simulink model "AcasXuClosedLoop_all"  (Simulink 10.0)
settings: Solver=VariableStepAuto  StartTime=0.0  StopTime=20  RelTol=1e-3
libraries: neural x14040, simulink x2
(part 13 of 534)

== system AcasXuClosedLoop_all/networks/Networks_m_2/AcasXu_5_2/Layer 5 ==
  "a{4} " : Inport  PortDimensions=50
      mask Display: e = 0.06;c1 = [120 230 180]/255;c2 = ([120 230 180]+0)/(256+128);c3 = ([120 230 180]+128)/(256+128);patch([0 1 1 0],[0 0 1 1],c1);patch([0 1 1 1-e 1-e 0+e],[0 0 1 1-e 0+e 0+e],c2);patch([0 0 1 1-e 0+e 0+e],[0 1 1 1-e 1-e 0+e],c3);plot([0 1 1 0 0],[0 0 1 1 0]);
  Delays 1 : Subsystem
      mask Display: e = 0.06;c1 = [120 150 230]/255;c2 = ([120 150 230]+0)/383;c3 = ([120 150 230]+128)/383;patch([0 1 1 0],[0 0 1 1],c1);patch([0 1 1 1-e 1-e 0+e],[0 0 1 1-e 0+e 0+e],c2);patch([0 0 1 1-e 0+e 0+e],[0 1 1 1-e 1-e 0+e],c3);plot([0 1 1 0 0],[0 0 1 1 0]);disp('0');
  "LW{5,4}" : Subsystem
      mask Display: e = 0.06;c1 = [120 150 230]/255;c2 = ([120 150 230]+0)/383;c3 = ([120 150 230]+128)/383;patch([0 1 1 0],[0 0 1 1],c1);patch([0 1 1 1-e 1-e 0+e],[0 0 1 1-e 0+e 0+e],c2);patch([0 0 1 1-e 0+e 0+e],[0 1 1 1-e 1-e 0+e],c3);plot([0 1 1 0 0],[0 0 1 1 0]);disp('W');
  "b{5}" : Constant  Value=[-0.913216999999999945458739603054709732532501220703125;0.09844060000000000310027559180525713600218296051025390625;1.1548899999999999721467247582040727138519287109375;-0.32563399999999997902477844036184251308441162109375;-0.1335260000000000057962523669630172662436962127685546875;0.341119000000000005545786052607581950724124908447265625;0.01802020000000000010675904604795505292713642120361328125;0.9002499999999999946709294817992486059665679931640625;0.18333399999999999696598251830437220633029937744140625;0.51134500000000004948930154569097794592380523681640625;-0.33117099999999999315747345463023521006107330322265625;0.8964060000000000361097818313282914459705352783203125;1.518850000000000033395508580724708735942840576171875;0.369159999999999988151699881200329400599002838134765625;-1.723759999999999958930629873066209256649017333984375;0.410592999999999985760723575367592275142669677734375;-0.186315000000000008384404281969182193279266357421875;0.265000999999999986567189580455305986106395721435546875;-0.1680520000000000069295680305003770627081394195556640625;0.198382000000000002781774810500792227685451507568359375;2.13187000000000015376144801848568022251129150390625;-0.058779299999999999604138878339654183946549892425537109375;0.64183900000000004837374945054762065410614013671875;-0.430126999999999981572074148061801679432392120361328125;0.022663200000000001399502735921487328596413135528564453125;-0.038571899999999999242294990153823164291679859161376953125;-0.1602760000000000017994494783124537207186222076416015625;0.32549499999999997879029933756100945174694061279296875;-0.49118600000000001148947603724082000553607940673828125;0.56853900000000001657696202528313733637332916259765625;-2.884020000000000027995383788947947323322296142578125;0.540777999999999980929032972198911011219024658203125;0.77833300000000005258726787360501475632190704345703125;-0.85023800000000004928324415232054889202117919921875;0.88560099999999997155697428752318955957889556884765625;-1.1629199999999999537436679020174778997898101806640625;-0.4910769999999999857465127206523902714252471923828125;0.689135999999999970810904414975084364414215087890625;-0.56418400000000001881517164292745292186737060546875;0.2479699999999999959765517587584326975047588348388671875;-0.38862900000000000222399876292911358177661895751953125;-0.04462950000000000250199860829525277949869632720947265625;0.4766340000000000021174173525650985538959503173828125;0.10841499999999999748201418015014496631920337677001953125;-1.9298900000000001053734877132228575646877288818359375;-1.2899700000000000610356210017926059663295745849609375;0.192620999999999986673770990819321013987064361572265625;-0.24735099999999998754418584212544374167919158935546875;0.300580000000000013837819778927951119840145111083984375;0.1147660000000000068975936073911725543439388275146484375]
      mask Display: e = 0.06;c1 = [120 150 230]/255;c2 = ([120 150 230]+0)/383;c3 = ([120 150 230]+128)/383;patch([0 1 1 0],[0 0 1 1],c1);patch([0 1 1 1-e 1-e 0+e],[0 0 1 1-e 0+e 0+e],c2);patch([0 0 1 1-e 0+e 0+e],[0 1 1 1-e 1-e 0+e],c3);plot([0 1 1 0 0],[0 0 1 1 0]);disp('b');
  netsum : Sum  Inputs=2  SaturateOnIntegerOverflow=on  InputSameDT=on  OutDataTypeStr=Inherit: Same as first input
      mask Display: e = 0.06;c1 = [120 150 230]/255;c2 = ([120 150 230]+0)/383;c3 = ([120 150 230]+128)/383;patch([0 1 1 0],[0 0 1 1],c1);patch([0 1 1 1-e 1-e 0+e],[0 0 1 1-e 0+e 0+e],c2);patch([0 0 1 1-e 0+e 0+e],[0 1 1 1-e 1-e 0+e],c3);plot([0 1 1 0 0],[0 0 1 1 0]);disp('+');
  poslin : neural/Transfer Functions/poslin
      settings: SourceProductName=Deep Learning Toolbox  SourceProductBaseCode=NN
  "a{5}" : Outport  InitialOutput=[0;0;0;0;0;0;0;0;0;0;0;0;0;0;0;0;0;0;0;0;0;0;0;0;0;0;0;0;0;0;0;0;0;0;0;0;0;0;0;0;0;0;0;0;0;0;0;0;0;0]
      mask Display: e = 0.06;c1 = [120 230 180]/255;c2 = ([120 230 180]+0)/(256+128);c3 = ([120 230 180]+128)/(256+128);patch([0 1 1 0],[0 0 1 1],c1);patch([0 1 1 1-e 1-e 0+e],[0 0 1 1-e 0+e 0+e],c2);patch([0 0 1 1-e 0+e 0+e],[0 1 1 1-e 1-e 0+e],c3);plot([0 1 1 0 0],[0 0 1 1 0]);
  wires:
  poslin/1 -> "a{5}"/1
  netsum/1 -> poslin/1
  "b{5}"/1 -> netsum/2
  "LW{5,4}"/1("lz{5,4}") -> netsum/1
  Delays 1/1("pd{5,4}") -> "LW{5,4}"/1("ad{5,4}")
  "a{4} "/1 -> Delays 1/1("p{5}")

== system AcasXuClosedLoop_all/networks/Networks_m_2/AcasXu_5_2/Layer 6 ==
  "a{5} " : Inport  PortDimensions=50
      mask Display: e = 0.06;c1 = [120 230 180]/255;c2 = ([120 230 180]+0)/(256+128);c3 = ([120 230 180]+128)/(256+128);patch([0 1 1 0],[0 0 1 1],c1);patch([0 1 1 1-e 1-e 0+e],[0 0 1 1-e 0+e 0+e],c2);patch([0 0 1 1-e 0+e 0+e],[0 1 1 1-e 1-e 0+e],c3);plot([0 1 1 0 0],[0 0 1 1 0]);
  Delays 1 : Subsystem
      mask Display: e = 0.06;c1 = [120 150 230]/255;c2 = ([120 150 230]+0)/383;c3 = ([120 150 230]+128)/383;patch([0 1 1 0],[0 0 1 1],c1);patch([0 1 1 1-e 1-e 0+e],[0 0 1 1-e 0+e 0+e],c2);patch([0 0 1 1-e 0+e 0+e],[0 1 1 1-e 1-e 0+e],c3);plot([0 1 1 0 0],[0 0 1 1 0]);disp('0');
  "LW{6,5}" : Subsystem
      mask Display: e = 0.06;c1 = [120 150 230]/255;c2 = ([120 150 230]+0)/383;c3 = ([120 150 230]+128)/383;patch([0 1 1 0],[0 0 1 1],c1);patch([0 1 1 1-e 1-e 0+e],[0 0 1 1-e 0+e 0+e],c2);patch([0 0 1 1-e 0+e 0+e],[0 1 1 1-e 1-e 0+e],c3);plot([0 1 1 0 0],[0 0 1 1 0]);disp('W');
  "b{6}" : Constant  Value=[-0.9019589999999999552215967923984862864017486572265625;-0.6001440000000000107860387288383208215236663818359375;0.006781279999999999773263592572902780375443398952484130859375;-0.572092000000000044934722609468735754489898681640625;0.08324209999999999942676964792553917504847049713134765625;-0.7174430000000000529070121046970598399639129638671875;-0.1013799999999999978950171453107031993567943572998046875;-3.266340000000000021174173525650985538959503173828125;0.07243670000000000663842314452267601154744625091552734375;-0.793306999999999984396481522708199918270111083984375;0.302810999999999996834532112188753671944141387939453125;-0.192081000000000001737276988933444954454898834228515625;-0.435099999999999986766141546468134038150310516357421875;-0.535789999999999988489207680686376988887786865234375;-0.57309299999999996355626308286446146667003631591796875;-0.2132600000000000051159076974727213382720947265625;-0.3339710000000000178488335222937166690826416015625;0.06419239999999999668478523062731255777180194854736328125;0.217891000000000001346478484265389852225780487060546875;0.288712999999999997413624441833235323429107666015625;0.1783079999999999942783546202917932532727718353271484375;0.2600569999999999826201246833079494535923004150390625;0.00048685299999999998195321371241561791975982487201690673828125;-0.54256000000000004224176564093795605003833770751953125;0.1129239999999999966018293662273208610713481903076171875;0.339301000000000019252155425419914536178112030029296875;-0.028156199999999999283861740195789025165140628814697265625;0.1899220000000000074802386507144547067582607269287109375;0.48247899999999999121058635864756070077419281005859375;-0.56484900000000004549605137071921490132808685302734375;-1.706630000000000091375795818748883903026580810546875;-0.06226299999999999890132329483094508759677410125732421875;0.42013299999999997869082335455459542572498321533203125;0.291520999999999974594544482897617854177951812744140625;0.0129844999999999995143884490289565292187035083770751953125;0.2454429999999999945092810094138258136808872222900390625;-0.37894200000000000105870867628254927694797515869140625;0.192157999999999995477395486886962316930294036865234375;0.26512999999999997680077967743272893130779266357421875;0.32366499999999998049560190338524989783763885498046875;0.169895999999999991469934457199997268617153167724609375;-0.24311599999999999877076106713502667844295501708984375;-0.90561700000000000532196509084315039217472076416015625;-0.54213900000000003753797273020609281957149505615234375;-1.55600000000000004973799150320701301097869873046875;0.398214999999999985647036737645976245403289794921875;0.213907999999999987039700499735772609710693359375;0.162391000000000007563727422166266478598117828369140625;0.518564000000000024925839170464314520359039306640625;0.4594329999999999802895445100148208439350128173828125]
      mask Display: e = 0.06;c1 = [120 150 230]/255;c2 = ([120 150 230]+0)/383;c3 = ([120 150 230]+128)/383;patch([0 1 1 0],[0 0 1 1],c1);patch([0 1 1 1-e 1-e 0+e],[0 0 1 1-e 0+e 0+e],c2);patch([0 0 1 1-e 0+e 0+e],[0 1 1 1-e 1-e 0+e],c3);plot([0 1 1 0 0],[0 0 1 1 0]);disp('b');
  netsum : Sum  Inputs=2  SaturateOnIntegerOverflow=on  InputSameDT=on  OutDataTypeStr=Inherit: Same as first input
      mask Display: e = 0.06;c1 = [120 150 230]/255;c2 = ([120 150 230]+0)/383;c3 = ([120 150 230]+128)/383;patch([0 1 1 0],[0 0 1 1],c1);patch([0 1 1 1-e 1-e 0+e],[0 0 1 1-e 0+e 0+e],c2);patch([0 0 1 1-e 0+e 0+e],[0 1 1 1-e 1-e 0+e],c3);plot([0 1 1 0 0],[0 0 1 1 0]);disp('+');
  poslin : neural/Transfer Functions/poslin
      settings: SourceProductName=Deep Learning Toolbox  SourceProductBaseCode=NN
  "a{6}" : Outport  InitialOutput=[0;0;0;0;0;0;0;0;0;0;0;0;0;0;0;0;0;0;0;0;0;0;0;0;0;0;0;0;0;0;0;0;0;0;0;0;0;0;0;0;0;0;0;0;0;0;0;0;0;0]
      mask Display: e = 0.06;c1 = [120 230 180]/255;c2 = ([120 230 180]+0)/(256+128);c3 = ([120 230 180]+128)/(256+128);patch([0 1 1 0],[0 0 1 1],c1);patch([0 1 1 1-e 1-e 0+e],[0 0 1 1-e 0+e 0+e],c2);patch([0 0 1 1-e 0+e 0+e],[0 1 1 1-e 1-e 0+e],c3);plot([0 1 1 0 0],[0 0 1 1 0]);
  wires:
  poslin/1 -> "a{6}"/1
  netsum/1 -> poslin/1
  "b{6}"/1 -> netsum/2
  "LW{6,5}"/1("lz{6,5}") -> netsum/1
  Delays 1/1("pd{6,5}") -> "LW{6,5}"/1("ad{6,5}")
  "a{5} "/1 -> Delays 1/1("p{6}")

== system AcasXuClosedLoop_all/networks/Networks_m_2/AcasXu_5_2/Layer 7 ==
  "a{6} " : Inport  PortDimensions=50
      mask Display: e = 0.06;c1 = [120 230 180]/255;c2 = ([120 230 180]+0)/(256+128);c3 = ([120 230 180]+128)/(256+128);patch([0 1 1 0],[0 0 1 1],c1);patch([0 1 1 1-e 1-e 0+e],[0 0 1 1-e 0+e 0+e],c2);patch([0 0 1 1-e 0+e 0+e],[0 1 1 1-e 1-e 0+e],c3);plot([0 1 1 0 0],[0 0 1 1 0]);
  Delays 1 : Subsystem
      mask Display: e = 0.06;c1 = [120 150 230]/255;c2 = ([120 150 230]+0)/383;c3 = ([120 150 230]+128)/383;patch([0 1 1 0],[0 0 1 1],c1);patch([0 1 1 1-e 1-e 0+e],[0 0 1 1-e 0+e 0+e],c2);patch([0 0 1 1-e 0+e 0+e],[0 1 1 1-e 1-e 0+e],c3);plot([0 1 1 0 0],[0 0 1 1 0]);disp('0');
  "LW{7,6}" : Subsystem
      mask Display: e = 0.06;c1 = [120 150 230]/255;c2 = ([120 150 230]+0)/383;c3 = ([120 150 230]+128)/383;patch([0 1 1 0],[0 0 1 1],c1);patch([0 1 1 1-e 1-e 0+e],[0 0 1 1-e 0+e 0+e],c2);patch([0 0 1 1-e 0+e 0+e],[0 1 1 1-e 1-e 0+e],c3);plot([0 1 1 0 0],[0 0 1 1 0]);disp('W');
  "b{7}" : Constant  Value=[-0.0170457999999999998408384271897375583648681640625;0.0262195999999999991569854529416261357255280017852783203125;-0.00996234000000000008423484132435987703502178192138671875;0.0286117000000000003268052495286610792391002178192138671875;-0.01021919999999999946471707090722702560015022754669189453125]
      mask Display: e = 0.06;c1 = [120 150 230]/255;c2 = ([120 150 230]+0)/383;c3 = ([120 150 230]+128)/383;patch([0 1 1 0],[0 0 1 1],c1);patch([0 1 1 1-e 1-e 0+e],[0 0 1 1-e 0+e 0+e],c2);patch([0 0 1 1-e 0+e 0+e],[0 1 1 1-e 1-e 0+e],c3);plot([0 1 1 0 0],[0 0 1 1 0]);disp('b');
  netsum : Sum  Inputs=2  SaturateOnIntegerOverflow=on  InputSameDT=on  OutDataTypeStr=Inherit: Same as first input
      mask Display: e = 0.06;c1 = [120 150 230]/255;c2 = ([120 150 230]+0)/383;c3 = ([120 150 230]+128)/383;patch([0 1 1 0],[0 0 1 1],c1);patch([0 1 1 1-e 1-e 0+e],[0 0 1 1-e 0+e 0+e],c2);patch([0 0 1 1-e 0+e 0+e],[0 1 1 1-e 1-e 0+e],c3);plot([0 1 1 0 0],[0 0 1 1 0]);disp('+');
  purelin : neural/Transfer Functions/purelin
      settings: SourceProductName=Deep Learning Toolbox
  "a{7}" : Outport  InitialOutput=[0;0;0;0;0]
      mask Display: e = 0.06;c1 = [120 230 180]/255;c2 = ([120 230 180]+0)/(256+128);c3 = ([120 230 180]+128)/(256+128);patch([0 1 1 0],[0 0 1 1],c1);patch([0 1 1 1-e 1-e 0+e],[0 0 1 1-e 0+e 0+e],c2);patch([0 0 1 1-e 0+e 0+e],[0 1 1 1-e 1-e 0+e],c3);plot([0 1 1 0 0],[0 0 1 1 0]);
  wires:
  purelin/1 -> "a{7}"/1
  netsum/1 -> purelin/1
  "b{7}"/1 -> netsum/2
  "LW{7,6}"/1("lz{7,6}") -> netsum/1
  Delays 1/1("pd{7,6}") -> "LW{7,6}"/1("ad{7,6}")
  "a{6} "/1 -> Delays 1/1("p{7}")

== system AcasXuClosedLoop_all/networks/Networks_m_2/AcasXu_5_2/Process Input 1 ==
  x : Inport  PortDimensions=5
      mask Display: e = 0.06;c1 = [120 230 180]/255;c2 = ([120 230 180]+0)/(256+128);c3 = ([120 230 180]+128)/(256+128);patch([0 1 1 0],[0 0 1 1],c1);patch([0 1 1 1-e 1-e 0+e],[0 0 1 1-e 0+e 0+e],c2);patch([0 0 1 1-e 0+e 0+e],[0 1 1 1-e 1-e 0+e],c3);plot([0 1 1 0 0],[0 0 1 1 0]);
  p : Outport  PortDimensions=5
      mask Display: e = 0.06;c1 = [120 230 180]/255;c2 = ([120 230 180]+0)/(256+128);c3 = ([120 230 180]+128)/(256+128);patch([0 1 1 0],[0 0 1 1],c1);patch([0 1 1 1-e 1-e 0+e],[0 0 1 1-e 0+e 0+e],c2);patch([0 0 1 1-e 0+e 0+e],[0 1 1 1-e 1-e 0+e],c3);plot([0 1 1 0 0],[0 0 1 1 0]);
  wires:
  x/1 -> p/1

== system AcasXuClosedLoop_all/networks/Networks_m_2/AcasXu_5_2/Process Output 1 ==
  a : Inport  PortDimensions=5
      mask Display: e = 0.06;c1 = [120 230 180]/255;c2 = ([120 230 180]+0)/(256+128);c3 = ([120 230 180]+128)/(256+128);patch([0 1 1 0],[0 0 1 1],c1);patch([0 1 1 1-e 1-e 0+e],[0 0 1 1-e 0+e 0+e],c2);patch([0 0 1 1-e 0+e 0+e],[0 1 1 1-e 1-e 0+e],c3);plot([0 1 1 0 0],[0 0 1 1 0]);
  y : Outport  PortDimensions=5
      mask Display: e = 0.06;c1 = [120 230 180]/255;c2 = ([120 230 180]+0)/(256+128);c3 = ([120 230 180]+128)/(256+128);patch([0 1 1 0],[0 0 1 1],c1);patch([0 1 1 1-e 1-e 0+e],[0 0 1 1-e 0+e 0+e],c2);patch([0 0 1 1-e 0+e 0+e],[0 1 1 1-e 1-e 0+e],c3);plot([0 1 1 0 0],[0 0 1 1 0]);
  wires:
  a/1 -> y/1

== system AcasXuClosedLoop_all/networks/Networks_m_3/AcasXu_1_3/Layer 1 ==
  "p{1}" : Inport  PortDimensions=5
      mask Display: e = 0.06;c1 = [120 230 180]/255;c2 = ([120 230 180]+0)/(256+128);c3 = ([120 230 180]+128)/(256+128);patch([0 1 1 0],[0 0 1 1],c1);patch([0 1 1 1-e 1-e 0+e],[0 0 1 1-e 0+e 0+e],c2);patch([0 0 1 1-e 0+e 0+e],[0 1 1 1-e 1-e 0+e],c3);plot([0 1 1 0 0],[0 0 1 1 0]);
  Delays 1 : Subsystem
      mask Display: e = 0.06;c1 = [120 150 230]/255;c2 = ([120 150 230]+0)/383;c3 = ([120 150 230]+128)/383;patch([0 1 1 0],[0 0 1 1],c1);patch([0 1 1 1-e 1-e 0+e],[0 0 1 1-e 0+e 0+e],c2);patch([0 0 1 1-e 0+e 0+e],[0 1 1 1-e 1-e 0+e],c3);plot([0 1 1 0 0],[0 0 1 1 0]);disp('0');
  "IW{1,1}" : Subsystem
      mask Display: e = 0.06;c1 = [120 150 230]/255;c2 = ([120 150 230]+0)/383;c3 = ([120 150 230]+128)/383;patch([0 1 1 0],[0 0 1 1],c1);patch([0 1 1 1-e 1-e 0+e],[0 0 1 1-e 0+e 0+e],c2);patch([0 0 1 1-e 0+e 0+e],[0 1 1 1-e 1-e 0+e],c3);plot([0 1 1 0 0],[0 0 1 1 0]);disp('W');
  "b{1}" : Constant  Value=[-0.1391890000000000071622707764618098735809326171875;0.31683600000000000651567688692011870443820953369140625;-0.0221072999999999998677058243856663466431200504302978515625;0.1543799999999999894573221581595134921371936798095703125;0.2399620000000000086259888121276162564754486083984375;-0.59694199999999997263699924587854184210300445556640625;-0.218791000000000013248069308247067965567111968994140625;-0.52023600000000003173994400640367530286312103271484375;-0.25257600000000002271605126225040294229984283447265625;-0.277005000000000001225686219186172820627689361572265625;0.0182437000000000015820234011698630638420581817626953125;0.0059112299999999999566835384712248924188315868377685546875;0.00655909999999999999975575093458246556110680103302001953125;0.0703985000000000027409186031945864669978618621826171875;-0.17136899999999999355537738665589131414890289306640625;0.346650999999999986922460948335356079041957855224609375;-0.055691200000000003200728571073341299779713153839111328125;-0.44257000000000001893596390800666995346546173095703125;-0.378821999999999992070343068917281925678253173828125;-0.57500899999999999234745473586372099816799163818359375;-0.338834999999999997299937604111619293689727783203125;0.08411349999999999382804816150382976047694683074951171875;-0.1049339999999999994972910144497291184961795806884765625;0.005628679999999999621074220357286321814171969890594482421875;-0.55048200000000002685140998437418602406978607177734375;0.14674799999999998956212721168412826955318450927734375;0.0878107999999999944140682828219723887741565704345703125;-0.026337699999999998612398854902494349516928195953369140625;0.1983959999999999890274438030246528796851634979248046875;0.3874750000000000138555833473219536244869232177734375;-0.019566699999999999370903225326401297934353351593017578125;0.0110390999999999996072919117295896285213530063629150390625;0.0252531999999999999528821348349083564244210720062255859375;-0.52901299999999995549160303198732435703277587890625;-0.0443296999999999996600053009387920610606670379638671875;0.0748424000000000033683278388707549311220645904541015625;-0.119566000000000005609734898825990967452526092529296875;-0.202324000000000003840483486783341504633426666259765625;-0.2654360000000000052722270993399433791637420654296875;0.142747000000000012764900247930199839174747467041015625;0.2083359999999999934150451963432715274393558502197265625;0.00851196000000000070617733882727407035417854785919189453125;-0.348104000000000024517277097402256913483142852783203125;-0.353781999999999985373477784378337673842906951904296875;-0.275040000000000006696865284538944251835346221923828125;-0.5273499999999999854338739169179461896419525146484375;0.046664299999999998835864545299045857973396778106689453125;0.0108835000000000008124612094206895562820136547088623046875;0.163235999999999992216004329748102463781833648681640625;0.10850600000000000522337728625643649138510227203369140625]
      mask Display: e = 0.06;c1 = [120 150 230]/255;c2 = ([120 150 230]+0)/383;c3 = ([120 150 230]+128)/383;patch([0 1 1 0],[0 0 1 1],c1);patch([0 1 1 1-e 1-e 0+e],[0 0 1 1-e 0+e 0+e],c2);patch([0 0 1 1-e 0+e 0+e],[0 1 1 1-e 1-e 0+e],c3);plot([0 1 1 0 0],[0 0 1 1 0]);disp('b');
  netsum : Sum  Inputs=2  SaturateOnIntegerOverflow=on  InputSameDT=on  OutDataTypeStr=Inherit: Same as first input
      mask Display: e = 0.06;c1 = [120 150 230]/255;c2 = ([120 150 230]+0)/383;c3 = ([120 150 230]+128)/383;patch([0 1 1 0],[0 0 1 1],c1);patch([0 1 1 1-e 1-e 0+e],[0 0 1 1-e 0+e 0+e],c2);patch([0 0 1 1-e 0+e 0+e],[0 1 1 1-e 1-e 0+e],c3);plot([0 1 1 0 0],[0 0 1 1 0]);disp('+');
  poslin : neural/Transfer Functions/poslin
      settings: SourceProductName=Deep Learning Toolbox  SourceProductBaseCode=NN
  "a{1}" : Outport  InitialOutput=[0;0;0;0;0;0;0;0;0;0;0;0;0;0;0;0;0;0;0;0;0;0;0;0;0;0;0;0;0;0;0;0;0;0;0;0;0;0;0;0;0;0;0;0;0;0;0;0;0;0]
      mask Display: e = 0.06;c1 = [120 230 180]/255;c2 = ([120 230 180]+0)/(256+128);c3 = ([120 230 180]+128)/(256+128);patch([0 1 1 0],[0 0 1 1],c1);patch([0 1 1 1-e 1-e 0+e],[0 0 1 1-e 0+e 0+e],c2);patch([0 0 1 1-e 0+e 0+e],[0 1 1 1-e 1-e 0+e],c3);plot([0 1 1 0 0],[0 0 1 1 0]);
  wires:
  poslin/1 -> "a{1}"/1
  netsum/1 -> poslin/1
  "b{1}"/1 -> netsum/2
  "IW{1,1}"/1("iz{1,1}") -> netsum/1
  Delays 1/1("pd{1,1}") -> "IW{1,1}"/1("pd{1,1}")
  "p{1}"/1 -> Delays 1/1("p{1}")

== system AcasXuClosedLoop_all/networks/Networks_m_3/AcasXu_1_3/Layer 2 ==
  "a{1} " : Inport  PortDimensions=50
      mask Display: e = 0.06;c1 = [120 230 180]/255;c2 = ([120 230 180]+0)/(256+128);c3 = ([120 230 180]+128)/(256+128);patch([0 1 1 0],[0 0 1 1],c1);patch([0 1 1 1-e 1-e 0+e],[0 0 1 1-e 0+e 0+e],c2);patch([0 0 1 1-e 0+e 0+e],[0 1 1 1-e 1-e 0+e],c3);plot([0 1 1 0 0],[0 0 1 1 0]);
  Delays 1 : Subsystem
      mask Display: e = 0.06;c1 = [120 150 230]/255;c2 = ([120 150 230]+0)/383;c3 = ([120 150 230]+128)/383;patch([0 1 1 0],[0 0 1 1],c1);patch([0 1 1 1-e 1-e 0+e],[0 0 1 1-e 0+e 0+e],c2);patch([0 0 1 1-e 0+e 0+e],[0 1 1 1-e 1-e 0+e],c3);plot([0 1 1 0 0],[0 0 1 1 0]);disp('0');
  "LW{2,1}" : Subsystem
      mask Display: e = 0.06;c1 = [120 150 230]/255;c2 = ([120 150 230]+0)/383;c3 = ([120 150 230]+128)/383;patch([0 1 1 0],[0 0 1 1],c1);patch([0 1 1 1-e 1-e 0+e],[0 0 1 1-e 0+e 0+e],c2);patch([0 0 1 1-e 0+e 0+e],[0 1 1 1-e 1-e 0+e],c3);plot([0 1 1 0 0],[0 0 1 1 0]);disp('W');
  "b{2}" : Constant  Value=[0.347727999999999981550757865988998673856258392333984375;0.541762999999999994571453498792834579944610595703125;0.1898910000000000042330583482907968573272228240966796875;0.479627999999999998781419208171428181231021881103515625;-0.473580999999999974203745978229562751948833465576171875;0.040631599999999996886135278373330947943031787872314453125;-0.8030049999999999688071739001316018402576446533203125;-0.2116139999999999965485386610453133471310138702392578125;0.07146850000000000424638102458629873581230640411376953125;0.042963200000000000000621724893790087662637233734130859375;0.4851039999999999796642669025459326803684234619140625;-0.007289740000000000282043277621824017842300236225128173828125;0.1341690000000000104751762819432769902050495147705078125;-0.01472270000000000002293720768875573412515223026275634765625;-0.1752559999999999951203477621675119735300540924072265625;-0.01234080000000000072735151235292505589313805103302001953125;0.1429999999999999882316359389733406715095043182373046875;0.0791975000000000040056846728475647978484630584716796875;-0.593301999999999996049382389173842966556549072265625;-0.01758579999999999865512023689007037319242954254150390625;-0.8927899999999999725019961260841228067874908447265625;0.284561000000000008380851568290381692349910736083984375;-0.364956000000000002625455408633570186793804168701171875;-0.008456180000000000640536512719336315058171749114990234375;-0.094960600000000006115641326687182299792766571044921875;0.1659460000000000101660901918876334093511104583740234375;0.1873650000000000037658764995285309851169586181640625;0.74341500000000004799716180059476755559444427490234375;-0.000422511999999999996076305297521003012661822140216827392578125;-0.0844598000000000015408119224957772530615329742431640625;-0.7734389999999999876223455430590547621250152587890625;0.03534790000000000154134482954759732820093631744384765625;0.490904000000000007020162229309789836406707763671875;0.145444999999999990958343687452725134789943695068359375;-0.17461599999999999344169054893427528440952301025390625;0.19807900000000000506616970596951432526111602783203125;-0.72643500000000005289990667733945883810520172119140625;-0.0070337400000000001309974351215714705176651477813720703125;0.452475000000000016076029396572266705334186553955078125;0.350580999999999975980102817629813216626644134521484375;0.210886999999999991128873944035149179399013519287109375;0.11957399999999999973265829567026230506598949432373046875;-0.433831000000000022165380642036325298249721527099609375;-0.0307224000000000004029221400969618116505444049835205078125;-0.395322000000000006725286993969348259270191192626953125;0.4742990000000000261337618212564848363399505615234375;0.1041640000000000065849548036567284725606441497802734375;0.56280699999999994620480947560281492769718170166015625;-0.66942900000000005178435458219610154628753662109375;-0.0213747999999999993558930100334691815078258514404296875]
      mask Display: e = 0.06;c1 = [120 150 230]/255;c2 = ([120 150 230]+0)/383;c3 = ([120 150 230]+128)/383;patch([0 1 1 0],[0 0 1 1],c1);patch([0 1 1 1-e 1-e 0+e],[0 0 1 1-e 0+e 0+e],c2);patch([0 0 1 1-e 0+e 0+e],[0 1 1 1-e 1-e 0+e],c3);plot([0 1 1 0 0],[0 0 1 1 0]);disp('b');
  netsum : Sum  Inputs=2  SaturateOnIntegerOverflow=on  InputSameDT=on  OutDataTypeStr=Inherit: Same as first input
      mask Display: e = 0.06;c1 = [120 150 230]/255;c2 = ([120 150 230]+0)/383;c3 = ([120 150 230]+128)/383;patch([0 1 1 0],[0 0 1 1],c1);patch([0 1 1 1-e 1-e 0+e],[0 0 1 1-e 0+e 0+e],c2);patch([0 0 1 1-e 0+e 0+e],[0 1 1 1-e 1-e 0+e],c3);plot([0 1 1 0 0],[0 0 1 1 0]);disp('+');
  poslin : neural/Transfer Functions/poslin
      settings: SourceProductName=Deep Learning Toolbox  SourceProductBaseCode=NN
  "a{2}" : Outport  InitialOutput=[0;0;0;0;0;0;0;0;0;0;0;0;0;0;0;0;0;0;0;0;0;0;0;0;0;0;0;0;0;0;0;0;0;0;0;0;0;0;0;0;0;0;0;0;0;0;0;0;0;0]
      mask Display: e = 0.06;c1 = [120 230 180]/255;c2 = ([120 230 180]+0)/(256+128);c3 = ([120 230 180]+128)/(256+128);patch([0 1 1 0],[0 0 1 1],c1);patch([0 1 1 1-e 1-e 0+e],[0 0 1 1-e 0+e 0+e],c2);patch([0 0 1 1-e 0+e 0+e],[0 1 1 1-e 1-e 0+e],c3);plot([0 1 1 0 0],[0 0 1 1 0]);
  wires:
  poslin/1 -> "a{2}"/1
  netsum/1 -> poslin/1
  "b{2}"/1 -> netsum/2
  "LW{2,1}"/1("lz{2,1}") -> netsum/1
  Delays 1/1("pd{2,1}") -> "LW{2,1}"/1("ad{2,1}")
  "a{1} "/1 -> Delays 1/1("p{2}")

== system AcasXuClosedLoop_all/networks/Networks_m_3/AcasXu_1_3/Layer 3 ==
  "a{2} " : Inport  PortDimensions=50
      mask Display: e = 0.06;c1 = [120 230 180]/255;c2 = ([120 230 180]+0)/(256+128);c3 = ([120 230 180]+128)/(256+128);patch([0 1 1 0],[0 0 1 1],c1);patch([0 1 1 1-e 1-e 0+e],[0 0 1 1-e 0+e 0+e],c2);patch([0 0 1 1-e 0+e 0+e],[0 1 1 1-e 1-e 0+e],c3);plot([0 1 1 0 0],[0 0 1 1 0]);
  Delays 1 : Subsystem
      mask Display: e = 0.06;c1 = [120 150 230]/255;c2 = ([120 150 230]+0)/383;c3 = ([120 150 230]+128)/383;patch([0 1 1 0],[0 0 1 1],c1);patch([0 1 1 1-e 1-e 0+e],[0 0 1 1-e 0+e 0+e],c2);patch([0 0 1 1-e 0+e 0+e],[0 1 1 1-e 1-e 0+e],c3);plot([0 1 1 0 0],[0 0 1 1 0]);disp('0');
  "LW{3,2}" : Subsystem
      mask Display: e = 0.06;c1 = [120 150 230]/255;c2 = ([120 150 230]+0)/383;c3 = ([120 150 230]+128)/383;patch([0 1 1 0],[0 0 1 1],c1);patch([0 1 1 1-e 1-e 0+e],[0 0 1 1-e 0+e 0+e],c2);patch([0 0 1 1-e 0+e 0+e],[0 1 1 1-e 1-e 0+e],c3);plot([0 1 1 0 0],[0 0 1 1 0]);disp('W');
  "b{3}" : Constant  Value=[-0.1647599999999999897948299576455610804259777069091796875;-0.63401300000000004875033710050047375261783599853515625;0.5627649999999999597122268824023194611072540283203125;-0.366914000000000017909229654833325184881687164306640625;-0.0142071000000000002838618229361600242555141448974609375;-1.485079999999999955662133288569748401641845703125;-0.288750000000000006661338147750939242541790008544921875;-0.171393999999999990802024285585503093898296356201171875;-0.1360929999999999917559279083434375934302806854248046875;0.03186909999999999743014456043965765275061130523681640625;-0.330610000000000014974688156144111417233943939208984375;-0.1883579999999999976534326151522691361606121063232421875;0.5184779999999999944293449516408145427703857421875;0.73671699999999995522870221975608728826045989990234375;0.2497230000000000005311306949806748889386653900146484375;0.405988999999999988776977488669217564165592193603515625;0.207932000000000005712763595511205494403839111328125;-1.15392999999999990023979989928193390369415283203125;-0.01751350000000000128874688698488171212375164031982421875;-0.78874400000000000066080474425689317286014556884765625;-0.70750100000000004651923291021375916898250579833984375;-0.57351399999999996826005599359632469713687896728515625;-0.253267999999999993132604458878631703555583953857421875;-0.70990399999999997948663121860590763390064239501953125;0.183537000000000005695000027117202989757061004638671875;-0.125307000000000001715960706860641948878765106201171875;-0.6910410000000000163566937771975062787532806396484375;0.329925999999999997047694932916783727705478668212890625;-0.01037330000000000022886137429622976924292743206024169921875;0.33924900000000002275868382639600895345211029052734375;-0.0282962999999999999245492432464743615128099918365478515625;-0.350183999999999995278443520874134264886379241943359375;-0.007730629999999999620030610714138674666173756122589111328125;-0.60501700000000002699351853152620606124401092529296875;-1.301050000000000039790393202565610408782958984375;0.6313180000000000458015847470960579812526702880859375;0.1850320000000000020268231537556857801973819732666015625;-0.59571300000000004803979436474037356674671173095703125;-2.37546999999999997044142219237983226776123046875;0.195614000000000010093259561472223140299320220947265625;0.3570670000000000232631691687856800854206085205078125;0.12270799999999999763122815465976600535213947296142578125;-0.93496400000000001728750476104323752224445343017578125;-0.1396880000000000066062710857295314781367778778076171875;0.66845699999999996787636291628587059676647186279296875;-0.0083978000000000004032774114648418617434799671173095703125;0.348939999999999972413178284114110283553600311279296875;-0.286432000000000019923618310713209211826324462890625;1.8850000000000000088817841970012523233890533447265625;-0.37483100000000002527400511098676361143589019775390625]
      mask Display: e = 0.06;c1 = [120 150 230]/255;c2 = ([120 150 230]+0)/383;c3 = ([120 150 230]+128)/383;patch([0 1 1 0],[0 0 1 1],c1);patch([0 1 1 1-e 1-e 0+e],[0 0 1 1-e 0+e 0+e],c2);patch([0 0 1 1-e 0+e 0+e],[0 1 1 1-e 1-e 0+e],c3);plot([0 1 1 0 0],[0 0 1 1 0]);disp('b');
  netsum : Sum  Inputs=2  SaturateOnIntegerOverflow=on  InputSameDT=on  OutDataTypeStr=Inherit: Same as first input
      mask Display: e = 0.06;c1 = [120 150 230]/255;c2 = ([120 150 230]+0)/383;c3 = ([120 150 230]+128)/383;patch([0 1 1 0],[0 0 1 1],c1);patch([0 1 1 1-e 1-e 0+e],[0 0 1 1-e 0+e 0+e],c2);patch([0 0 1 1-e 0+e 0+e],[0 1 1 1-e 1-e 0+e],c3);plot([0 1 1 0 0],[0 0 1 1 0]);disp('+');
  poslin : neural/Transfer Functions/poslin
      settings: SourceProductName=Deep Learning Toolbox  SourceProductBaseCode=NN
  "a{3}" : Outport  InitialOutput=[0;0;0;0;0;0;0;0;0;0;0;0;0;0;0;0;0;0;0;0;0;0;0;0;0;0;0;0;0;0;0;0;0;0;0;0;0;0;0;0;0;0;0;0;0;0;0;0;0;0]
      mask Display: e = 0.06;c1 = [120 230 180]/255;c2 = ([120 230 180]+0)/(256+128);c3 = ([120 230 180]+128)/(256+128);patch([0 1 1 0],[0 0 1 1],c1);patch([0 1 1 1-e 1-e 0+e],[0 0 1 1-e 0+e 0+e],c2);patch([0 0 1 1-e 0+e 0+e],[0 1 1 1-e 1-e 0+e],c3);plot([0 1 1 0 0],[0 0 1 1 0]);
  wires:
  poslin/1 -> "a{3}"/1
  netsum/1 -> poslin/1
  "b{3}"/1 -> netsum/2
  "LW{3,2}"/1("lz{3,2}") -> netsum/1
  Delays 1/1("pd{3,2}") -> "LW{3,2}"/1("ad{3,2}")
  "a{2} "/1 -> Delays 1/1("p{3}")

== system AcasXuClosedLoop_all/networks/Networks_m_3/AcasXu_1_3/Layer 4 ==
  "a{3} " : Inport  PortDimensions=50
      mask Display: e = 0.06;c1 = [120 230 180]/255;c2 = ([120 230 180]+0)/(256+128);c3 = ([120 230 180]+128)/(256+128);patch([0 1 1 0],[0 0 1 1],c1);patch([0 1 1 1-e 1-e 0+e],[0 0 1 1-e 0+e 0+e],c2);patch([0 0 1 1-e 0+e 0+e],[0 1 1 1-e 1-e 0+e],c3);plot([0 1 1 0 0],[0 0 1 1 0]);
  Delays 1 : Subsystem
      mask Display: e = 0.06;c1 = [120 150 230]/255;c2 = ([120 150 230]+0)/383;c3 = ([120 150 230]+128)/383;patch([0 1 1 0],[0 0 1 1],c1);patch([0 1 1 1-e 1-e 0+e],[0 0 1 1-e 0+e 0+e],c2);patch([0 0 1 1-e 0+e 0+e],[0 1 1 1-e 1-e 0+e],c3);plot([0 1 1 0 0],[0 0 1 1 0]);disp('0');
  "LW{4,3}" : Subsystem
      mask Display: e = 0.06;c1 = [120 150 230]/255;c2 = ([120 150 230]+0)/383;c3 = ([120 150 230]+128)/383;patch([0 1 1 0],[0 0 1 1],c1);patch([0 1 1 1-e 1-e 0+e],[0 0 1 1-e 0+e 0+e],c2);patch([0 0 1 1-e 0+e 0+e],[0 1 1 1-e 1-e 0+e],c3);plot([0 1 1 0 0],[0 0 1 1 0]);disp('W');
  "b{4}" : Constant  Value=[-0.2037440000000000084323659166329889558255672454833984375;1.2988299999999999290167806975659914314746856689453125;-0.7242509999999999781294945933041162788867950439453125;0.1195360000000000033626434969846741296350955963134765625;0.019295500000000000151434420558871352113783359527587890625;-0.54689200000000004475708692552871070802211761474609375;-0.693130000000000023874235921539366245269775390625;-0.051194700000000002704236834460971294902265071868896484375;-1.1275299999999999212008106042048893868923187255859375;-1.6139200000000000212452277992269955575466156005859375;0.287161999999999972832398498212569393217563629150390625;-0.30206699999999997441335608527879230678081512451171875;-0.42720000000000002415845301584340631961822509765625;-0.273635000000000017106316363424411974847316741943359375;1.0479700000000000681410483593936078250408172607421875;-0.0236182999999999983231635525271485676057636737823486328125;-0.474412000000000000365929508916451595723628997802734375;0.11025100000000000177724501781995058991014957427978515625;0.324483000000000021412205342130619101226329803466796875;0.431923999999999974619413478649221360683441162109375;-0.5913779999999999592574795315158553421497344970703125;4.44188000000000027256419343757443130016326904296875;-0.53031700000000003836220230368780903518199920654296875;-0.10392200000000000048583359557596850208938121795654296875;-0.056534199999999999730615485304952017031610012054443359375;-2.17147999999999985476506481063552200794219970703125;-0.3075959999999999805453398948884569108486175537109375;-0.70530199999999998450306293307221494615077972412109375;0.1765279999999999904769509839752572588622570037841796875;-0.9987909999999999843822706679929979145526885986328125;-0.220712999999999992528643133482546545565128326416015625;0.466955000000000008952838470577262341976165771484375;-1.471060000000000034248159863636828958988189697265625;-0.030357399999999999662403382671982399187982082366943359375;-0.376931999999999989281462831058888696134090423583984375;0.23735899999999998666311284978291951119899749755859375;-0.43797099999999999919708670859108678996562957763671875;-0.251454000000000010839329434020328335464000701904296875;-0.0180718999999999983818721460693268454633653163909912109375;-0.12060099999999999986766141546468134038150310516357421875;-0.9861400000000000165556457432103343307971954345703125;-0.08018069999999999364348468589014373719692230224609375;-1.19948999999999994514610079932026565074920654296875;1.3087400000000000144240175359300337731838226318359375;-1.648179999999999978399500832892954349517822265625;0.7158670000000000310791392621467821300029754638671875;-0.1435970000000000024176216584237408824265003204345703125;0.0340306999999999970629716017356258817017078399658203125;-0.05425649999999999917310589125918340869247913360595703125;-1.221109999999999917719151198980398476123809814453125]
      mask Display: e = 0.06;c1 = [120 150 230]/255;c2 = ([120 150 230]+0)/383;c3 = ([120 150 230]+128)/383;patch([0 1 1 0],[0 0 1 1],c1);patch([0 1 1 1-e 1-e 0+e],[0 0 1 1-e 0+e 0+e],c2);patch([0 0 1 1-e 0+e 0+e],[0 1 1 1-e 1-e 0+e],c3);plot([0 1 1 0 0],[0 0 1 1 0]);disp('b');
  netsum : Sum  Inputs=2  SaturateOnIntegerOverflow=on  InputSameDT=on  OutDataTypeStr=Inherit: Same as first input
      mask Display: e = 0.06;c1 = [120 150 230]/255;c2 = ([120 150 230]+0)/383;c3 = ([120 150 230]+128)/383;patch([0 1 1 0],[0 0 1 1],c1);patch([0 1 1 1-e 1-e 0+e],[0 0 1 1-e 0+e 0+e],c2);patch([0 0 1 1-e 0+e 0+e],[0 1 1 1-e 1-e 0+e],c3);plot([0 1 1 0 0],[0 0 1 1 0]);disp('+');
  poslin : neural/Transfer Functions/poslin
      settings: SourceProductName=Deep Learning Toolbox  SourceProductBaseCode=NN
  "a{4}" : Outport  InitialOutput=[0;0;0;0;0;0;0;0;0;0;0;0;0;0;0;0;0;0;0;0;0;0;0;0;0;0;0;0;0;0;0;0;0;0;0;0;0;0;0;0;0;0;0;0;0;0;0;0;0;0]
      mask Display: e = 0.06;c1 = [120 230 180]/255;c2 = ([120 230 180]+0)/(256+128);c3 = ([120 230 180]+128)/(256+128);patch([0 1 1 0],[0 0 1 1],c1);patch([0 1 1 1-e 1-e 0+e],[0 0 1 1-e 0+e 0+e],c2);patch([0 0 1 1-e 0+e 0+e],[0 1 1 1-e 1-e 0+e],c3);plot([0 1 1 0 0],[0 0 1 1 0]);
  wires:
  poslin/1 -> "a{4}"/1
  netsum/1 -> poslin/1
  "b{4}"/1 -> netsum/2
  "LW{4,3}"/1("lz{4,3}") -> netsum/1
  Delays 1/1("pd{4,3}") -> "LW{4,3}"/1("ad{4,3}")
  "a{3} "/1 -> Delays 1/1("p{4}")

== system AcasXuClosedLoop_all/networks/Networks_m_3/AcasXu_1_3/Layer 5 ==
  "a{4} " : Inport  PortDimensions=50
      mask Display: e = 0.06;c1 = [120 230 180]/255;c2 = ([120 230 180]+0)/(256+128);c3 = ([120 230 180]+128)/(256+128);patch([0 1 1 0],[0 0 1 1],c1);patch([0 1 1 1-e 1-e 0+e],[0 0 1 1-e 0+e 0+e],c2);patch([0 0 1 1-e 0+e 0+e],[0 1 1 1-e 1-e 0+e],c3);plot([0 1 1 0 0],[0 0 1 1 0]);
  Delays 1 : Subsystem
      mask Display: e = 0.06;c1 = [120 150 230]/255;c2 = ([120 150 230]+0)/383;c3 = ([120 150 230]+128)/383;patch([0 1 1 0],[0 0 1 1],c1);patch([0 1 1 1-e 1-e 0+e],[0 0 1 1-e 0+e 0+e],c2);patch([0 0 1 1-e 0+e 0+e],[0 1 1 1-e 1-e 0+e],c3);plot([0 1 1 0 0],[0 0 1 1 0]);disp('0');
  "LW{5,4}" : Subsystem
      mask Display: e = 0.06;c1 = [120 150 230]/255;c2 = ([120 150 230]+0)/383;c3 = ([120 150 230]+128)/383;patch([0 1 1 0],[0 0 1 1],c1);patch([0 1 1 1-e 1-e 0+e],[0 0 1 1-e 0+e 0+e],c2);patch([0 0 1 1-e 0+e 0+e],[0 1 1 1-e 1-e 0+e],c3);plot([0 1 1 0 0],[0 0 1 1 0]);disp('W');
  "b{5}" : Constant  Value=[-0.343893000000000004234834705130197107791900634765625;1.8282199999999999562305674771778285503387451171875;-2.367649999999999810285089552053250372409820556640625;0.00055711699999999998549060631347629168885760009288787841796875;-0.7545960000000000444941861132974736392498016357421875;-1.035849999999999937472239253111183643341064453125;2.26897999999999999687361196265555918216705322265625;0.2302589999999999914592763161635957658290863037109375;-0.0132186999999999998944844037396251223981380462646484375;0.283376000000000016765255850259563885629177093505859375;0.5390859999999999541131501246127299964427947998046875;0.75770800000000004814637577510438859462738037109375;-0.6985369999999999635065250913612544536590576171875;-0.4432139999999999968594011079403571784496307373046875;-1.99063999999999996504129740060307085514068603515625;-1.122009999999999951825202515465207397937774658203125;-2.131200000000000205346850634668953716754913330078125;-0.12669900000000000606092953603365458548069000244140625;-0.50494099999999997319122257977141998708248138427734375;-1.714869999999999894413349466049112379550933837890625;-1.4968399999999999483435431102407164871692657470703125;2.9002900000000000346744855050928890705108642578125;-1.617410000000000014352963262354023754596710205078125;-2.303020000000000067075234255753457546234130859375;-0.350818999999999991956656231195665895938873291015625;-1.98622999999999994003019310184754431247711181640625;1.99195000000000010942358130705542862415313720703125;-1.568319999999999936335370875895023345947265625;-4.3266299999999997538679963327012956142425537109375;-3.210210000000000007958078640513122081756591796875;-0.26744800000000001904965074572828598320484161376953125;0.194732999999999989437782232926110737025737762451171875;2.994479999999999808579786986229009926319122314453125;0.88611899999999999000266370785539038479328155517578125;-0.9040660000000000362518903784803114831447601318359375;1.6914100000000000800781663201632909476757049560546875;1.085809999999999941877604214823804795742034912109375;-1.1222499999999999698019337301957421004772186279296875;0.1353999999999999925837101955039543099701404571533203125;0.9661880000000000467963445771601982414722442626953125;-2.205979999999999829896069059032015502452850341796875;-2.297940000000000093649532573181204497814178466796875;0.9408760000000000456310544905136339366436004638671875;0.3270399999999999973709918776876293122768402099609375;-0.624138999999999999346300683100707828998565673828125;-0.92281299999999999439381781485280953347682952880859375;0.422352000000000005197620112085132859647274017333984375;-1.035179999999999989057641869294457137584686279296875;-0.676892000000000049197979024029336869716644287109375;0.431369999999999975681674868610571138560771942138671875]
      mask Display: e = 0.06;c1 = [120 150 230]/255;c2 = ([120 150 230]+0)/383;c3 = ([120 150 230]+128)/383;patch([0 1 1 0],[0 0 1 1],c1);patch([0 1 1 1-e 1-e 0+e],[0 0 1 1-e 0+e 0+e],c2);patch([0 0 1 1-e 0+e 0+e],[0 1 1 1-e 1-e 0+e],c3);plot([0 1 1 0 0],[0 0 1 1 0]);disp('b');
  netsum : Sum  Inputs=2  SaturateOnIntegerOverflow=on  InputSameDT=on  OutDataTypeStr=Inherit: Same as first input
      mask Display: e = 0.06;c1 = [120 150 230]/255;c2 = ([120 150 230]+0)/383;c3 = ([120 150 230]+128)/383;patch([0 1 1 0],[0 0 1 1],c1);patch([0 1 1 1-e 1-e 0+e],[0 0 1 1-e 0+e 0+e],c2);patch([0 0 1 1-e 0+e 0+e],[0 1 1 1-e 1-e 0+e],c3);plot([0 1 1 0 0],[0 0 1 1 0]);disp('+');
  poslin : neural/Transfer Functions/poslin
      settings: SourceProductName=Deep Learning Toolbox  SourceProductBaseCode=NN
  "a{5}" : Outport  InitialOutput=[0;0;0;0;0;0;0;0;0;0;0;0;0;0;0;0;0;0;0;0;0;0;0;0;0;0;0;0;0;0;0;0;0;0;0;0;0;0;0;0;0;0;0;0;0;0;0;0;0;0]
      mask Display: e = 0.06;c1 = [120 230 180]/255;c2 = ([120 230 180]+0)/(256+128);c3 = ([120 230 180]+128)/(256+128);patch([0 1 1 0],[0 0 1 1],c1);patch([0 1 1 1-e 1-e 0+e],[0 0 1 1-e 0+e 0+e],c2);patch([0 0 1 1-e 0+e 0+e],[0 1 1 1-e 1-e 0+e],c3);plot([0 1 1 0 0],[0 0 1 1 0]);
  wires:
  poslin/1 -> "a{5}"/1
  netsum/1 -> poslin/1
  "b{5}"/1 -> netsum/2
  "LW{5,4}"/1("lz{5,4}") -> netsum/1
  Delays 1/1("pd{5,4}") -> "LW{5,4}"/1("ad{5,4}")
  "a{4} "/1 -> Delays 1/1("p{5}")

== system AcasXuClosedLoop_all/networks/Networks_m_3/AcasXu_1_3/Layer 6 ==
  "a{5} " : Inport  PortDimensions=50
      mask Display: e = 0.06;c1 = [120 230 180]/255;c2 = ([120 230 180]+0)/(256+128);c3 = ([120 230 180]+128)/(256+128);patch([0 1 1 0],[0 0 1 1],c1);patch([0 1 1 1-e 1-e 0+e],[0 0 1 1-e 0+e 0+e],c2);patch([0 0 1 1-e 0+e 0+e],[0 1 1 1-e 1-e 0+e],c3);plot([0 1 1 0 0],[0 0 1 1 0]);
  Delays 1 : Subsystem
      mask Display: e = 0.06;c1 = [120 150 230]/255;c2 = ([120 150 230]+0)/383;c3 = ([120 150 230]+128)/383;patch([0 1 1 0],[0 0 1 1],c1);patch([0 1 1 1-e 1-e 0+e],[0 0 1 1-e 0+e 0+e],c2);patch([0 0 1 1-e 0+e 0+e],[0 1 1 1-e 1-e 0+e],c3);plot([0 1 1 0 0],[0 0 1 1 0]);disp('0');
  "LW{6,5}" : Subsystem
      mask Display: e = 0.06;c1 = [120 150 230]/255;c2 = ([120 150 230]+0)/383;c3 = ([120 150 230]+128)/383;patch([0 1 1 0],[0 0 1 1],c1);patch([0 1 1 1-e 1-e 0+e],[0 0 1 1-e 0+e 0+e],c2);patch([0 0 1 1-e 0+e 0+e],[0 1 1 1-e 1-e 0+e],c3);plot([0 1 1 0 0],[0 0 1 1 0]);disp('W');
  "b{6}" : Constant  Value=[0.94024600000000002619771066747489385306835174560546875;1.4219699999999999562305674771778285503387451171875;0.91954100000000005277200898490264080464839935302734375;1.1900999999999999356958824137109331786632537841796875;1.5567200000000001036681851473986171185970306396484375;1.0074099999999999166533370953402481973171234130859375;-1.9777100000000000790123522165231406688690185546875;-1.40678999999999998493649400188587605953216552734375;-1.2098199999999998954791635696892626583576202392578125;1.535369999999999901518776823650114238262176513671875;-0.63651000000000002021494083237485028803348541259765625;0.1227229999999999987547738555804244242608547210693359375;0.503492000000000050619064495549537241458892822265625;0.90856999999999998873789763820241205394268035888671875;0.960231999999999974448883222066797316074371337890625;1.8264199999999999324273858292144723236560821533203125;-1.22036999999999995480948200565762817859649658203125;0.6921910000000000007247535904753021895885467529296875;0.93729600000000001802646920623374171555042266845703125;1.5334399999999999142374917937559075653553009033203125;-3.164149999999999796074234836851246654987335205078125;-1.76944999999999996731503415503539144992828369140625;2.11373999999999995225152815692126750946044921875;0.71909299999999998220800989656709134578704833984375;-2.44930000000000003268496584496460855007171630859375;-0.07358499999999999763300451149916625581681728363037109375;2.326709999999999833875108379288576543331146240234375;0.7065099999999999713651277488679625093936920166015625;-1.5062699999999999977973175191436894237995147705078125;1.0603800000000001002575800157501362264156341552734375;0.54418800000000000505195885125431232154369354248046875;0.900495999999999963137042868766002357006072998046875;-0.374406000000000016569856597925536334514617919921875;0.154778999999999999914734871708787977695465087890625;0.4039960000000000217568185689742676913738250732421875;0.183321000000000011720402426362852565944194793701171875;-1.300289999999999945856643535080365836620330810546875;1.451480000000000103455022326670587062835693359375;1.679910000000000014352963262354023754596710205078125;1.3920999999999998930633182681049220263957977294921875;-0.04102440000000000253077558909353683702647686004638671875;-1.7685800000000000409272615797817707061767578125;1.062559999999999948983031572424806654453277587890625;0.32273600000000002285815980940242297947406768798828125;0.6643280000000000296012103717657737433910369873046875;0.419883000000000006224354365258477628231048583984375;1.1733700000000000240873987422673963010311126708984375;0.2601240000000000218705054066958837211132049560546875;-0.006586119999999999995166088950782068422995507717132568359375;1.3007500000000000728306304154102690517902374267578125]
      mask Display: e = 0.06;c1 = [120 150 230]/255;c2 = ([120 150 230]+0)/383;c3 = ([120 150 230]+128)/383;patch([0 1 1 0],[0 0 1 1],c1);patch([0 1 1 1-e 1-e 0+e],[0 0 1 1-e 0+e 0+e],c2);patch([0 0 1 1-e 0+e 0+e],[0 1 1 1-e 1-e 0+e],c3);plot([0 1 1 0 0],[0 0 1 1 0]);disp('b');
  netsum : Sum  Inputs=2  SaturateOnIntegerOverflow=on  InputSameDT=on  OutDataTypeStr=Inherit: Same as first input
      mask Display: e = 0.06;c1 = [120 150 230]/255;c2 = ([120 150 230]+0)/383;c3 = ([120 150 230]+128)/383;patch([0 1 1 0],[0 0 1 1],c1);patch([0 1 1 1-e 1-e 0+e],[0 0 1 1-e 0+e 0+e],c2);patch([0 0 1 1-e 0+e 0+e],[0 1 1 1-e 1-e 0+e],c3);plot([0 1 1 0 0],[0 0 1 1 0]);disp('+');
  poslin : neural/Transfer Functions/poslin
      settings: SourceProductName=Deep Learning Toolbox  SourceProductBaseCode=NN
  "a{6}" : Outport  InitialOutput=[0;0;0;0;0;0;0;0;0;0;0;0;0;0;0;0;0;0;0;0;0;0;0;0;0;0;0;0;0;0;0;0;0;0;0;0;0;0;0;0;0;0;0;0;0;0;0;0;0;0]
      mask Display: e = 0.06;c1 = [120 230 180]/255;c2 = ([120 230 180]+0)/(256+128);c3 = ([120 230 180]+128)/(256+128);patch([0 1 1 0],[0 0 1 1],c1);patch([0 1 1 1-e 1-e 0+e],[0 0 1 1-e 0+e 0+e],c2);patch([0 0 1 1-e 0+e 0+e],[0 1 1 1-e 1-e 0+e],c3);plot([0 1 1 0 0],[0 0 1 1 0]);
  wires:
  poslin/1 -> "a{6}"/1
  netsum/1 -> poslin/1
  "b{6}"/1 -> netsum/2
  "LW{6,5}"/1("lz{6,5}") -> netsum/1
  Delays 1/1("pd{6,5}") -> "LW{6,5}"/1("ad{6,5}")
  "a{5} "/1 -> Delays 1/1("p{6}")

== system AcasXuClosedLoop_all/networks/Networks_m_3/AcasXu_1_3/Layer 7 ==
  "a{6} " : Inport  PortDimensions=50
      mask Display: e = 0.06;c1 = [120 230 180]/255;c2 = ([120 230 180]+0)/(256+128);c3 = ([120 230 180]+128)/(256+128);patch([0 1 1 0],[0 0 1 1],c1);patch([0 1 1 1-e 1-e 0+e],[0 0 1 1-e 0+e 0+e],c2);patch([0 0 1 1-e 0+e 0+e],[0 1 1 1-e 1-e 0+e],c3);plot([0 1 1 0 0],[0 0 1 1 0]);
  Delays 1 : Subsystem
      mask Display: e = 0.06;c1 = [120 150 230]/255;c2 = ([120 150 230]+0)/383;c3 = ([120 150 230]+128)/383;patch([0 1 1 0],[0 0 1 1],c1);patch([0 1 1 1-e 1-e 0+e],[0 0 1 1-e 0+e 0+e],c2);patch([0 0 1 1-e 0+e 0+e],[0 1 1 1-e 1-e 0+e],c3);plot([0 1 1 0 0],[0 0 1 1 0]);disp('0');
  "LW{7,6}" : Subsystem
      mask Display: e = 0.06;c1 = [120 150 230]/255;c2 = ([120 150 230]+0)/383;c3 = ([120 150 230]+128)/383;patch([0 1 1 0],[0 0 1 1],c1);patch([0 1 1 1-e 1-e 0+e],[0 0 1 1-e 0+e 0+e],c2);patch([0 0 1 1-e 0+e 0+e],[0 1 1 1-e 1-e 0+e],c3);plot([0 1 1 0 0],[0 0 1 1 0]);disp('W');
  "b{7}" : Constant  Value=[-0.01377570000000000009776623954849128494970500469207763671875;-0.019359700000000000519317921998663223348557949066162109375;-0.01979430000000000078319573049157042987644672393798828125;-0.019322499999999999398259120653165155090391635894775390625;-0.0189473999999999996590727135981069295667111873626708984375]
      mask Display: e = 0.06;c1 = [120 150 230]/255;c2 = ([120 150 230]+0)/383;c3 = ([120 150 230]+128)/383;patch([0 1 1 0],[0 0 1 1],c1);patch([0 1 1 1-e 1-e 0+e],[0 0 1 1-e 0+e 0+e],c2);patch([0 0 1 1-e 0+e 0+e],[0 1 1 1-e 1-e 0+e],c3);plot([0 1 1 0 0],[0 0 1 1 0]);disp('b');
  netsum : Sum  Inputs=2  SaturateOnIntegerOverflow=on  InputSameDT=on  OutDataTypeStr=Inherit: Same as first input
      mask Display: e = 0.06;c1 = [120 150 230]/255;c2 = ([120 150 230]+0)/383;c3 = ([120 150 230]+128)/383;patch([0 1 1 0],[0 0 1 1],c1);patch([0 1 1 1-e 1-e 0+e],[0 0 1 1-e 0+e 0+e],c2);patch([0 0 1 1-e 0+e 0+e],[0 1 1 1-e 1-e 0+e],c3);plot([0 1 1 0 0],[0 0 1 1 0]);disp('+');
  purelin : neural/Transfer Functions/purelin
      settings: SourceProductName=Deep Learning Toolbox
  "a{7}" : Outport  InitialOutput=[0;0;0;0;0]
      mask Display: e = 0.06;c1 = [120 230 180]/255;c2 = ([120 230 180]+0)/(256+128);c3 = ([120 230 180]+128)/(256+128);patch([0 1 1 0],[0 0 1 1],c1);patch([0 1 1 1-e 1-e 0+e],[0 0 1 1-e 0+e 0+e],c2);patch([0 0 1 1-e 0+e 0+e],[0 1 1 1-e 1-e 0+e],c3);plot([0 1 1 0 0],[0 0 1 1 0]);
  wires:
  purelin/1 -> "a{7}"/1
  netsum/1 -> purelin/1
  "b{7}"/1 -> netsum/2
  "LW{7,6}"/1("lz{7,6}") -> netsum/1
  Delays 1/1("pd{7,6}") -> "LW{7,6}"/1("ad{7,6}")
  "a{6} "/1 -> Delays 1/1("p{7}")

== system AcasXuClosedLoop_all/networks/Networks_m_3/AcasXu_1_3/Process Input 1 ==
  x : Inport  PortDimensions=5
      mask Display: e = 0.06;c1 = [120 230 180]/255;c2 = ([120 230 180]+0)/(256+128);c3 = ([120 230 180]+128)/(256+128);patch([0 1 1 0],[0 0 1 1],c1);patch([0 1 1 1-e 1-e 0+e],[0 0 1 1-e 0+e 0+e],c2);patch([0 0 1 1-e 0+e 0+e],[0 1 1 1-e 1-e 0+e],c3);plot([0 1 1 0 0],[0 0 1 1 0]);
  p : Outport  PortDimensions=5
      mask Display: e = 0.06;c1 = [120 230 180]/255;c2 = ([120 230 180]+0)/(256+128);c3 = ([120 230 180]+128)/(256+128);patch([0 1 1 0],[0 0 1 1],c1);patch([0 1 1 1-e 1-e 0+e],[0 0 1 1-e 0+e 0+e],c2);patch([0 0 1 1-e 0+e 0+e],[0 1 1 1-e 1-e 0+e],c3);plot([0 1 1 0 0],[0 0 1 1 0]);
  wires:
  x/1 -> p/1
